simulink model "r2_ratty2_32k_r203"  (R2012b)
settings: Solver=ode45  StartTime=0.0  StopTime=10.0  RelTol=1e-3
undefined symbols (not defined in the file): Down, pfb_coeff_gen_calc, Up
libraries: xbsIndex_r4 x20024, casper_library_misc x89, casper_library_delays x41, xps_library x14, xbsReplacements_r4 x2, simulink x1, casper_library_scopes x1
(part 133 of 207)

== system r2_ratty2_32k_r203/fft/fft_direct/butterfly2_2/twiddle/coeff_gen/cosin ==
  theta : Inport
  misci : Inport  Port=2
  Constant4 : xbsIndex_r4/Constant  const=0  gui_display_data_type=Fixed-point  arith_type=Unsigned  n_bits=1  bin_pt=0  preci_type=Single  exp_width=8  frac_width=24  explicit_period=on  period=1  equ=P=C  opselect=C  inp2=PCIN>>17  opr=+  inp1=P  carry=CIN  dbl_ovrd=off  block_type=constant
  Constant5 : xbsIndex_r4/Constant  const=0  gui_display_data_type=Fixed-point  arith_type=Unsigned  n_bits=1  bin_pt=0  preci_type=Single  exp_width=8  frac_width=24  explicit_period=on  period=1  equ=P=C  opselect=C  inp2=PCIN>>17  opr=+  inp1=P  carry=CIN  dbl_ovrd=off  block_type=constant
  Delay : xbsIndex_r4/Delay  rst=off  infoeditControl=Selection of Reset will increase slice count due to use of real FFs and instead of SRLs  en=off  latency=bram_latency  dbl_ovrd=off  reg_retiming=on  block_type=delay
  Delay10 : xbsIndex_r4/Delay  rst=off  infoeditControl=Selection of Reset will increase slice count due to use of real FFs and instead of SRLs  en=off  latency=bram_latency  dbl_ovrd=off  reg_retiming=on  block_type=delay
  Delay8 : xbsIndex_r4/Delay  rst=off  infoeditControl=Selection of Reset will increase slice count due to use of real FFs and instead of SRLs  en=off  latency=bram_latency  dbl_ovrd=off  reg_retiming=on  block_type=delay
  ROM : xbsIndex_r4/ROM  depth=2^(13)  initVector=[24296199670 24293840365 24291481060 24289121755 24286762451 24284403146 24282305985 24279946680 24277587375 24275228070 24272868765 24270509460 24268150156 24265790851 24263431546 24261334385 24258975080 24256615775 24254256470 24251897165 24249537861 24247178556 24244819251 24242459946 24240100641 24237741336 24235644175 24233284870 24230925566 24228566261 24226206956 24223847651 24221488346 24219129041 24216769736 24214410432 24212051127 24209953966 24207594661 24205235356 24202876051 24200516747 24198157442 24195798137 24193438832 24191079527 24188720222 24186360917 24184001613 24181642308 24179545147 24177185842 24174826537 24172467232 24170107928 24167748623 24165389318 24163030013 24160670708 24158311403 24155952098 24153592794 24151233489 24148874184 24146514879 24144417718 24142058413 24139699109 24137339804 24134980499 24132621194 24130261889 24127902585 24125543280 24123183975 24120824670 24118465365 24116106060 24113746756 24111387451 24109028146 24106668841 24104309536 24101950232 24099590927 24097493766 24095134461 24092775156 24090415851 24088056547 24085697242 24083337937 24080978632 24078619327 24076260023 24073900718 24071541413 24069182108 24066822803 24064463499 24062104194 24059744889 24057385584 24055026279 24052666975 24050307670 24047948365 24045589060 24043229755 24040870451 24038511146 24036151841 24033792536 24031433231 24029073927 24026714622 24024355317 24021996012 24019636708 24017277403 24014918098 24012820937 24010461632 24008102328 24005743023 24003383718 24001024413 23998665109 23996305804 23993946499 23991587194 23989227889 23986868585 23984509280 23982149975 23979790670 23977431366 23975072061 23972712756 23970353451 23967994146 23965634842 23963275537 23960916232 23958556927 23956197623 23953838318 23951479013 23949119708 23946760404 23944138955 23941779650 23939420345 23937061041 23934701736 23932342431 23929983126 23927623822 23925264517 23922905212 23920545907 23918186603 23915827298 23913467993 23911108688 23908749384 23906390079 23904030774 23901671469 23899312165 23896952860 23894593555 23892234250 23889874946 23887515641 23885156336 23882797031 23880437727 23878078422 23875719117 23873359813 23871000508 23868641203 23866281898 23863922594 23861563289 23858941840 23856582535 23854223231 23851863926 23849504621 23847145317 23844786012 23842426707 23840067402 23837708098 23835348793 23832989488 23830630184 23828270879 23825911574 23823552269 23821192965 23818833660 23816474355 23814115051 23811493602 23809134297 23806774992 23804415688 23802056383 23799697078 23797337774 23794978469 23792619164 23790259859 23787900555 23785541250 23783181945 23780822641 23778463336 23775841887 23773482583 23771123278 23768763973 23766404669 23764045364 23761686059 23759326754 23756967450 23754608145 23752248840 23749889536 23747530231 23744908782 23742549478 23740190173 23737830868 23735471564 23733112259 23730752954 23728393650 23726034345 23723675040 23721315736 23718956431 23716334982 23713975678 23711616373 23709257068 23706897763 23704538459 23702179154 23699819849 23697460545 23695101240 23692479791 23690120487 23687761182 23685401877 23683042573 23680683268 23678323964 23675964659 23673605354 23671246050 23668624601 23666265296 23663905992 23661546687 23659187382 23656828078 23654468773 23652109468 23649750164 23647128715 23644769410 23642410106 23640050801 23637691496 23635332192 23632972887 23630613582 23627992134 23625632829 23623273525 23620914220 23618554915 23616195611 23613836306 23611477001 23608855553 23606496248 23604136943 23601777639 23599418334 23597059030 23594699725 23592078276 23589718972 23587359667 23585000362 23582641058 23580281753 23577922449 23575563144 23572941695 23570582391 23568223086 23565863781 23563504477 23561145172 23558523724 23556164419 23553805114 23551445810 23549086505 23546727201 23544367896 23541746447 23539387143 23537027838 23534668533 23532309229 23529949924 23527590620 23524969171 23522609866 23520250562 23517891257 23515531953 23513172648 23510551199 ...(+90893 chars)]  distributed_mem=Block RAM  rst=off  init_reg=0  en=off  latency=bram_latency  gui_display_data_type=Fixed-point  arith_type=Unsigned  n_bits=n_bits*2  bin_pt=0  preci_type=Single  exp_width=8  frac_width=24  dbl_ovrd=off  optimize=Speed  use_rpm=on  block_type=sprom
  Terminator : Terminator
  Terminator1 : Terminator
  Terminator4 : Terminator
  add_convert0 : Subsystem
  add_convert1 : Subsystem
  assert : xbsIndex_r4/Assert  assert_type=on  type_source=Explicitly  gui_display_data_type=Fixed-point  arith_type=Unsigned  n_bits=13  bin_pt=0  preci_type=Single  exp_width=8  frac_width=24  assert_rate=off  rate_source=Explicitly  period=1  output_port=on  block_type=assert
  c_to_ri : casper_library_misc/c_to_ri  n_bits=n_bits  bin_pt=bin_pt
  invert0 : Subsystem  mask{n_bits=n_bits, bin_pt=bin_pt, neg_latency=neg_latency, mux_latency=mux_latency}
      mask prompts: n_bits "bit width"; bin_pt "binary point"; neg_latency "negate latency"; mux_latency "mux latency"
  invert1 : Subsystem  mask{n_bits=n_bits, bin_pt=bin_pt, neg_latency=neg_latency, mux_latency=mux_latency}
      mask prompts: n_bits "bit width"; bin_pt "binary point"; neg_latency "negate latency"; mux_latency "mux latency"
  cos : Outport
  -sin : Outport  Port=2
  misco : Outport  Port=3
  wires:
  theta/1 -> assert/1
  assert/1 -> add_convert0/1(theta), add_convert1/1(theta)
  Constant4/1 -> add_convert0/2(misci)
  add_convert0/3(misco) -> Terminator/1
  misci/1 -> add_convert1/2(misci)
  add_convert1/3(misco) -> Delay/1
  add_convert0/2(add) -> ROM/1
  add_convert1/2(add) -> Terminator4/1
  ROM/1 -> c_to_ri/1
  add_convert1/1(negate) -> Delay8/1
  add_convert0/1(negate) -> Delay10/1
  Delay10/1 -> invert0/1(negate)
  Constant5/1 -> invert0/3(misci)
  c_to_ri/1 -> invert0/2(in)
  Delay8/1 -> invert1/1(negate)
  c_to_ri/2 -> invert1/2(in)
  invert0/2(misco) -> Terminator1/1
  Delay/1 -> invert1/3(misci)
  invert0/1(out) -> cos/1
  invert1/2(misco) -> misco/1
  invert1/1(out) -> -sin/1
